simulink model "robot_kinematic_sim"  (R2020b)
settings: Solver=VariableStepAuto  StartTime=0.0  StopTime=10.0  RelTol=1e-3
model workspace (MAT data in the model file):
  dq0 = [0; 0; 0; 0; 0; 0]
  q0 = [0; 0; 0; 0; 0; 0]
  robotToTest : robotics.RigidBodyTree (value not decoded)
undefined symbols (not defined in the file): X, Y, Z
libraries: fl_lib x114, sm_lib x104, nesl_utility x59, fl_ps_legacy_lib x48, ee_lib x12, simulink_extras x10, simulink x6
(part 1 of 5)
settings Model: Creator=jeason  LastModifiedBy=Administrator
settings ExplorerConfigSet: InternalSimMechanicsExplorerSettings="<ExplorerConfiguration><canonicalview>default</canonicalview><AnimationSetting><BasePlaybackSpeed>1.0</BasePlaybackSpeed><PlaybackSpeedFactor>1.0</PlaybackSpeedFactor></AnimationSetting><TilePanel><TileInfo><Tiles Columns=\"1\" Count=\"1\" Rows=\"1\"><Column Weight=\"1\"/><Row Weight=\"1\"/><Tile Height=\"1\" Width=\"1\" X=\"0\" Y=\"0\"/></Tiles></TileInfo><SceneInfo><SceneViewer><BGColor><R>0.800000011920928955</R><G>0.800000011920928955</G><B>0.800000011920928955</B></BGColor><Camera><DoPerspective>off</DoPerspective><Position><X>0.99999999731974798</X><Y>-0.0550754879096279348</Y><Z>0.380291677713366028</Z></Position><Center><X>-2.68025202032617926e-09</X><Y>-0.0550754879096279348</Y><Z>0.380291677713366028</Z></Center><Up><X>-0</X><Y>0</Y><Z>1</Z></Up><ViewLimDist>1</ViewLimDist><YLim>0.484052245622873412</YLim><XLim>0.721172433512456745</XLim></Camera></SceneViewer></SceneInfo></TilePanel><MechEditorPanel><Cameras/><GfxPanelCameraMap/><VideoCreator><PlaybackSpeedRatio>1.0</PlaybackSpeedRatio><FrameRate>30</FrameRate><VideoFormat>Motion JPEG AVI</VideoFormat><FrameSize>auto</FrameSize></VideoCreator></MechEditorPanel></ExplorerConfiguration>"

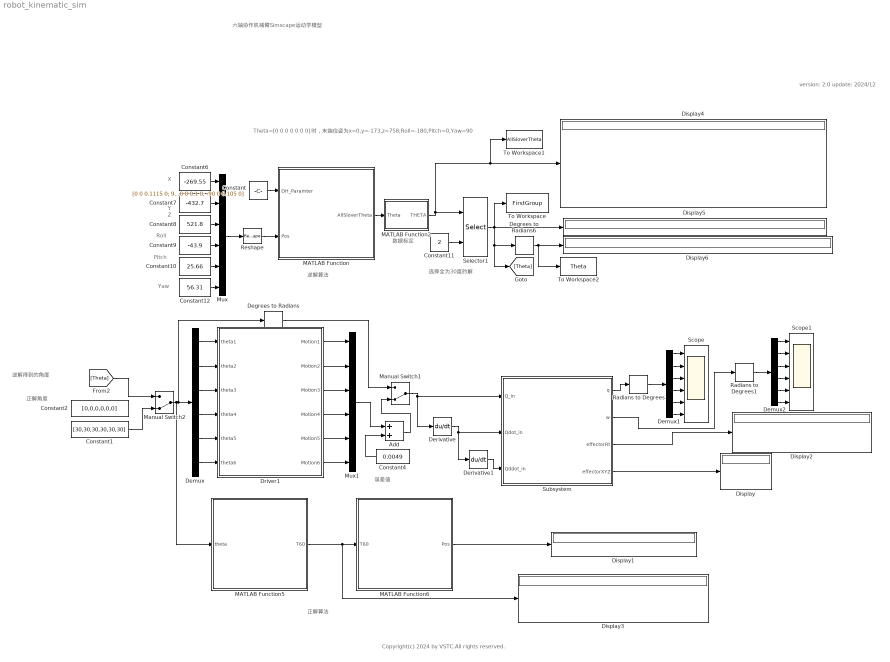
== system robot_kinematic_sim ==
  note: 六轴协作机械臂Simscape运动学模型
  note: Copyright(c) 2024 by VSTC.All rights reserved.
  note: Y
  note: Pitch
  note: X
  note: Theta=[0 0 0 0 0 0 0]时，末端位姿为x=0,y=-173,z=758;Roll=-180,Pitch=0,Yaw=90
  note: 数据标定
  note: Z
  note: 逆解算法
  note: Roll
  note: 选择全为30度的解
  note: Yaw
  note: 逆解得到的角度
  note: 正解角度
  note: 正解算法
  note: 误差值
  note: version: 2.0
      | update: 2024/12
  Add : Sum
  Constant : Constant  Value=[0 0 0.1115 0; 90 0 0 -90;0 -0.27 0 0; 0 -0.248 0.149 -90; 90 0 0.1 0; -90 0 0.105 0]
  Constant1 : Constant  Value=[30,30,30,30,30,30]
  Constant10 : Constant  Value=25.66
  Constant11 : Constant  Value=2
  Constant12 : Constant  Value=56.31
  Constant2 : Constant  Value=[0,0,0,0,0,0]
  Constant4 : Constant  Value=0.0049
  Constant6 : Constant  Value=-269.55
  Constant7 : Constant  Value=-432.7
  Constant8 : Constant  Value=521.8
  Constant9 : Constant  Value=-43.9
  Degrees to Radians : simulink_extras/Transformations/Degrees to Radians
  Degrees to Radians6 : simulink_extras/Transformations/Degrees to Radians
  Demux : Demux  Outputs=6
  Demux1 : Demux  Outputs=6
  Demux2 : Demux  Outputs=6
  Derivative : Derivative
  Derivative1 : Derivative
  Display{,1..6} (7 alike) : Display
  Driver1 : Subsystem  mask{angle1=0, angle2=0, angle3=0, angle4=0, angle5=0, angle6=0}
      mask prompts: angle1 "InitTheta1(deg)"; angle2 "InitTheta2(deg)"; angle3 "InitTheta3(deg)"; angle4 "InitTheta4(deg)"; angle5 "InitTheta5(deg)"; angle6 "InitTheta6(deg)"
  MATLAB Function : MATLAB Function  TreatAsAtomicUnit=on
  MATLAB Function2 : MATLAB Function  TreatAsAtomicUnit=on
  MATLAB Function5 : MATLAB Function  TreatAsAtomicUnit=on
  MATLAB Function6 : MATLAB Function  TreatAsAtomicUnit=on
  Manual Switch1 : ManualSwitch  CurrentSetting=0
  Manual Switch2 : ManualSwitch  CurrentSetting=0
  Mux : Mux  Inputs=6
  Mux1 : Mux  Inputs=6
  Radians to Degrees : simulink_extras/Transformations/Radians to Degrees
  Radians to Degrees1 : simulink_extras/Transformations/Radians to Degrees
  Reshape : Reshape  OutputDimensionality=Customize  OutputDimensions=[1,6]
  Scope : Scope  NumInputPorts=6
  Scope1 : Scope  NumInputPorts=6
  Selector1 : Selector  NumberOfDimensions=2  InputPortWidth=10  IndexOptions=Index vector (port),Starting index (dialog)  Indices=[1 3],1  OutputSizes=1,6
  Subsystem : Subsystem
  To Workspace : ToWorkspace  VariableName=FirstGroup  SampleTime=-1
      settings: MaxDataPoints=1
  To Workspace1 : ToWorkspace  VariableName=AllSloverTheta  SampleTime=-1
      settings: MaxDataPoints=1
  To Workspace2 : ToWorkspace  VariableName=Theta  SampleTime=-1
      settings: MaxDataPoints=1
  wires:
  Constant4/1 -> Add/2
  Degrees to Radians/1 -> Manual Switch1/1
  Driver1/6(Motion6) -> Mux1/6
  Manual Switch1/1 -> Derivative/1, Subsystem/1(Q_in)
  Demux1/6 -> Scope/6
  Driver1/5(Motion5) -> Mux1/5
  Degrees to Radians6/1 -> Display6/1, To Workspace2/1
  Demux/3 -> Driver1/3(theta3)
  Driver1/4(Motion4) -> Mux1/4
  Derivative1/1 -> Subsystem/3(Qddot_in)
  Constant12/1 -> Mux/6
  Demux/6 -> Driver1/6(theta6)
  Demux/4 -> Driver1/4(theta4)
  Driver1/3(Motion3) -> Mux1/3
  Driver1/2(Motion2) -> Mux1/2
  Constant/1 -> MATLAB Function/1(DH_Paramter)
  Demux1/1 -> Scope/1
  Driver1/1(Motion1) -> Mux1/1
  [Theta] -> Manual Switch2/1
  MATLAB Function6/1(Pos) -> Display1/1
  Demux/5 -> Driver1/5(theta5)
  Constant8/1 -> Mux/3
  Constant11/1 -> Selector1/2
  Demux/1 -> Driver1/1(theta1)
  MATLAB Function/1(AllSloverTheta) -> MATLAB Function2/1(Theta)
  Demux1/5 -> Scope/5
  Derivative/1 -> Derivative1/1, Subsystem/2(Qdot_in)
  Constant10/1 -> Mux/5
  Reshape/1 -> MATLAB Function/2(Pos)
  Demux1/3 -> Scope/3
  Constant7/1 -> Mux/2
  MATLAB Function2/1(THETA) -> Selector1/1, Display4/1, To Workspace1/1
  Constant6/1 -> Mux/1
  Constant9/1 -> Mux/4
  Selector1/1 -> Display5/1, Degrees to Radians6/1, [Theta], To Workspace/1
  Manual Switch2/1 -> MATLAB Function5/1(theta), Degrees to Radians/1, Demux/1
  Mux/1 -> Reshape/1
  Subsystem/4(effectorXYZ) -> Display/1
  Radians to Degrees/1 -> Demux1/1
  Demux1/4 -> Scope/4
  Subsystem/3(effectorRt) -> Display2/1
  Demux1/2 -> Scope/2
  Demux/2 -> Driver1/2(theta2)
  Demux2/2 -> Scope1/2
  Demux2/4 -> Scope1/4
  Radians to Degrees1/1 -> Demux2/1
  Demux2/3 -> Scope1/3
  Demux2/1 -> Scope1/1
  Subsystem/2(w) -> Radians to Degrees1/1
  Demux2/5 -> Scope1/5
  Demux2/6 -> Scope1/6
  Mux1/1 -> Add/1
  Add/1 -> Manual Switch1/2
  Subsystem/1(q) -> Radians to Degrees/1
  MATLAB Function5/1(T60) -> Display3/1, MATLAB Function6/1(T60)
  Constant1/1 -> Manual Switch2/2

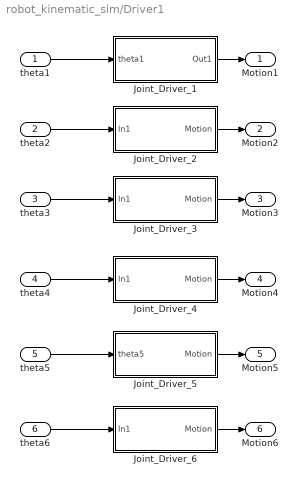
== system robot_kinematic_sim/Driver1 ==
  theta1 : Inport
  theta2 : Inport  Port=2
  theta3 : Inport  Port=3
  theta4 : Inport  Port=4
  theta5 : Inport  Port=5
  theta6 : Inport  Port=6
  Joint_Driver_1 : Subsystem
  Joint_Driver_2 : Subsystem
  Joint_Driver_3 : Subsystem
  Joint_Driver_4 : Subsystem
  Joint_Driver_5 : Subsystem
  Joint_Driver_6 : Subsystem
  Motion1 : Outport
  Motion2 : Outport  Port=2
  Motion3 : Outport  Port=3
  Motion4 : Outport  Port=4
  Motion5 : Outport  Port=5
  Motion6 : Outport  Port=6
  wires:
  theta2/1 -> Joint_Driver_2/1(In1)
  theta3/1 -> Joint_Driver_3/1(In1)
  theta4/1 -> Joint_Driver_4/1(In1)
  theta6/1 -> Joint_Driver_6/1(In1)
  theta1/1 -> Joint_Driver_1/1(theta1)
  Joint_Driver_1/1(Out1) -> Motion1/1
  Joint_Driver_2/1(Motion) -> Motion2/1
  Joint_Driver_3/1(Motion) -> Motion3/1
  Joint_Driver_4/1(Motion) -> Motion4/1
  Joint_Driver_5/1(Motion) -> Motion5/1
  Joint_Driver_6/1(Motion) -> Motion6/1
  theta5/1 -> Joint_Driver_5/1(theta5)

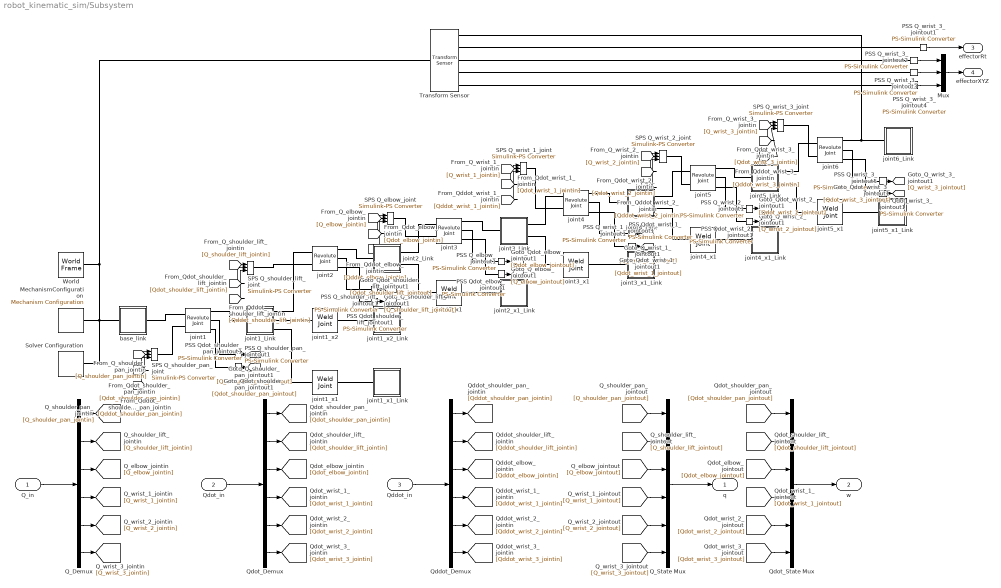
== system robot_kinematic_sim/Subsystem ==
  Q_in : Inport
  Qdot_in : Inport  Port=2
  Qddot_in : Inport  Port=3
  MechanismConfiguration : sm_lib/Utilities/Mechanism Configuration  UniformGravity=Constant  GravityVector=[0 0 -9.80665]  GravityVectorUnits=m/s^2  GravityUnits=m/s^2  LinearizationDelta=0.001  JointModeTransitionNonlinearIterations=2
  Mux : Mux  Inputs=3
  PSS Q_elbow_jointout1 : nesl_utility/PS-Simulink Converter  PhysicalDomain=network_engine_domain  SubClassName=ps_output  LeftPortType=input  RightPortType=output  PseudoPeriodic=off  Frequencies=[]  Unit=inherit  AffineConversion=off
  PSS Q_shoulder_lift_jointout1 : nesl_utility/PS-Simulink Converter  PhysicalDomain=network_engine_domain  SubClassName=ps_output  LeftPortType=input  RightPortType=output  PseudoPeriodic=off  Frequencies=[]  Unit=inherit  AffineConversion=off
  PSS Q_shoulder_pan_jointout1 : nesl_utility/PS-Simulink Converter  PhysicalDomain=network_engine_domain  SubClassName=ps_output  LeftPortType=input  RightPortType=output  PseudoPeriodic=off  Frequencies=[]  Unit=inherit  AffineConversion=off
  PSS Q_wrist_1_jointout1 : nesl_utility/PS-Simulink Converter  PhysicalDomain=network_engine_domain  SubClassName=ps_output  LeftPortType=input  RightPortType=output  PseudoPeriodic=off  Frequencies=[]  Unit=inherit  AffineConversion=off
  PSS Q_wrist_2_jointout1 : nesl_utility/PS-Simulink Converter  PhysicalDomain=network_engine_domain  SubClassName=ps_output  LeftPortType=input  RightPortType=output  PseudoPeriodic=off  Frequencies=[]  Unit=inherit  AffineConversion=off
  PSS Q_wrist_3_jointout{1..5} (5 alike) : nesl_utility/PS-Simulink Converter  PhysicalDomain=network_engine_domain  SubClassName=ps_output  LeftPortType=input  RightPortType=output  PseudoPeriodic=off  Frequencies=[]  Unit=inherit  AffineConversion=off
  PSS Qdot_elbow_jointout1 : nesl_utility/PS-Simulink Converter  PhysicalDomain=network_engine_domain  SubClassName=ps_output  LeftPortType=input  RightPortType=output  PseudoPeriodic=off  Frequencies=[]  Unit=inherit  AffineConversion=off
  PSS Qdot_shoulder_lift_jointout1 : nesl_utility/PS-Simulink Converter  PhysicalDomain=network_engine_domain  SubClassName=ps_output  LeftPortType=input  RightPortType=output  PseudoPeriodic=off  Frequencies=[]  Unit=inherit  AffineConversion=off
  PSS Qdot_shoulder_pan_jointout1 : nesl_utility/PS-Simulink Converter  PhysicalDomain=network_engine_domain  SubClassName=ps_output  LeftPortType=input  RightPortType=output  PseudoPeriodic=off  Frequencies=[]  Unit=inherit  AffineConversion=off
  PSS Qdot_wrist_1_jointout1 : nesl_utility/PS-Simulink Converter  PhysicalDomain=network_engine_domain  SubClassName=ps_output  LeftPortType=input  RightPortType=output  PseudoPeriodic=off  Frequencies=[]  Unit=inherit  AffineConversion=off
  PSS Qdot_wrist_2_jointout1 : nesl_utility/PS-Simulink Converter  PhysicalDomain=network_engine_domain  SubClassName=ps_output  LeftPortType=input  RightPortType=output  PseudoPeriodic=off  Frequencies=[]  Unit=inherit  AffineConversion=off
  PSS Qdot_wrist_3_jointout1 : nesl_utility/PS-Simulink Converter  PhysicalDomain=network_engine_domain  SubClassName=ps_output  LeftPortType=input  RightPortType=output  PseudoPeriodic=off  Frequencies=[]  Unit=inherit  AffineConversion=off
  Q_Demux : Demux  Outputs=6
  Q_State Mux : Mux  Inputs=6
  Qddot_Demux : Demux  Outputs=6
  Qdot_Demux : Demux  Outputs=6
  Qdot_State Mux : Mux  Inputs=6
  SPS Q_elbow_joint : nesl_utility/Simulink-PS Converter  PhysicalDomain=network_engine_domain  SubClassName=ps_input  LeftPortType=input  RightPortType=output  PseudoPeriodic=off  Frequencies=[]  NoiseDistribution=none  NoiseParameters=[]  Unit=1  AffineConversion=off  FilteringAndDerivatives=provide  SimscapeFilterOrder=1  InputFilterTimeConstant=0.001  UdotUserProvided=2
  SPS Q_shoulder_lift_joint : nesl_utility/Simulink-PS Converter  PhysicalDomain=network_engine_domain  SubClassName=ps_input  LeftPortType=input  RightPortType=output  PseudoPeriodic=off  Frequencies=[]  NoiseDistribution=none  NoiseParameters=[]  Unit=1  AffineConversion=off  FilteringAndDerivatives=provide  SimscapeFilterOrder=1  InputFilterTimeConstant=0.001  UdotUserProvided=2
  SPS Q_shoulder_pan_joint : nesl_utility/Simulink-PS Converter  PhysicalDomain=network_engine_domain  SubClassName=ps_input  LeftPortType=input  RightPortType=output  PseudoPeriodic=off  Frequencies=[]  NoiseDistribution=none  NoiseParameters=[]  Unit=1  AffineConversion=off  FilteringAndDerivatives=provide  SimscapeFilterOrder=1  InputFilterTimeConstant=0.001  UdotUserProvided=2
  SPS Q_wrist_1_joint : nesl_utility/Simulink-PS Converter  PhysicalDomain=network_engine_domain  SubClassName=ps_input  LeftPortType=input  RightPortType=output  PseudoPeriodic=off  Frequencies=[]  NoiseDistribution=none  NoiseParameters=[]  Unit=1  AffineConversion=off  FilteringAndDerivatives=provide  SimscapeFilterOrder=1  InputFilterTimeConstant=0.001  UdotUserProvided=2
  SPS Q_wrist_2_joint : nesl_utility/Simulink-PS Converter  PhysicalDomain=network_engine_domain  SubClassName=ps_input  LeftPortType=input  RightPortType=output  PseudoPeriodic=off  Frequencies=[]  NoiseDistribution=none  NoiseParameters=[]  Unit=1  AffineConversion=off  FilteringAndDerivatives=provide  SimscapeFilterOrder=1  InputFilterTimeConstant=0.001  UdotUserProvided=2
  SPS Q_wrist_3_joint : nesl_utility/Simulink-PS Converter  PhysicalDomain=network_engine_domain  SubClassName=ps_input  LeftPortType=input  RightPortType=output  PseudoPeriodic=off  Frequencies=[]  NoiseDistribution=none  NoiseParameters=[]  Unit=1  AffineConversion=off  FilteringAndDerivatives=provide  SimscapeFilterOrder=1  InputFilterTimeConstant=0.001  UdotUserProvided=2
  Solver Configuration : nesl_utility/Solver Configuration  PhysicalDomain=network_engine_domain  SubClassName=solver  LeftPortType=input  RightPortType=generic  Tones=[]  Harmonics=[]  SimulateNoise=off  Profile=off  UseLocalSampling=off  EnableSwitchedLinearOptims=on  FrequencyDomain=off  UseCCode=off  RelTol=0.001  AbsTol=1e-06  MinStep=1e-09  ToWorkspace=off  RevertToSquareIcSolve=off  NumHomotopyIterations=100  PhasorMode=off  PhasorModeNumHarmonics=1  DoDC=off  ResidualTolerance=1e-09  UseLocalSolver=off  LocalSolverChoice=NE_BACKWARD_EULER_ADVANCER  LocalSolverSampleTime=0.001  DoFixedCost=off  MaxNonlinIter=3  MaxModeIter=2  ComputeImpulses=off  ImpulseIterations=2  LinearAlgebra=auto  EquationFormulation=NE_TIME_EF  DelaysMemoryBudget=1024  AutomaticFiltering=on  FilteringTimeConstant=0.001  PartitionStorageMethod=AS_NEEDED  PartitionMemoryBudget=1024  PartitionMethod=ROBUST
  Transform Sensor : sm_lib/Frames and Transforms/Transform Sensor  MeasurementFrame=Base  SenseAngle=off  SenseAxis=off  SenseAngle1=off  SenseAngle2=off  SenseAngle3=off  SenseQ=off  SenseR=on  SenseOmegaX=off  SenseOmegaY=off  SenseOmegaZ=off  SenseOmega1=off  SenseOmega2=off  SenseOmega3=off  SenseQDot=off  SenseRDot=off  SenseAlphaX=off  SenseAlphaY=off  SenseAlphaZ=off  SenseAlpha1=off  SenseAlpha2=off  SenseAlpha3=off  SenseQDDot=off  SenseRDDot=off  SenseX=on  SenseY=on  SenseZ=on  SenseRad=off  SenseAzim=off  SenseDist=off  SenseInc=off  SenseXDot=off  SenseYDot=off  SenseZDot=off  SenseRadDot=off  SenseAzimDot=off  SenseDistDot=off  SenseIncDot=off  SenseXDDot=off  SenseYDDot=off  SenseZDDot=off  SenseRadDDot=off  SenseAzimDDot=off  SenseDistDDot=off  SenseIncDDot=off
  World : sm_lib/Frames and Transforms/World Frame
  base_link : Subsystem
  joint{1..6} (6 alike) : sm_lib/Joints/Revolute Joint  JointMode=Normal  SenseConstraintForce=off  SenseConstraintForceX=off  SenseConstraintForceY=off  SenseConstraintForceZ=off  SenseConstraintTorque=off  SenseConstraintTorqueX=off  SenseConstraintTorqueY=off  SenseConstraintTorqueZ=off  SenseTotalForce=off  SenseTotalForceX=off  SenseTotalForceY=off  SenseTotalForceZ=off  SenseTotalTorque=off  SenseTotalTorqueX=off  SenseTotalTorqueY=off  SenseTotalTorqueZ=off  CompositeWrenchDir=FollowerOnBase  CompositeWrenchFrame=BaseFrame  EquilibriumPosition=0  EquilibriumPositionUnits=deg  SpringStiffness=0  SpringStiffnessUnits=N*m/deg  DampingCoefficient=0  DampingCoefficientUnits=m^2*kg/s/rad  TorqueActuationMode=ComputedTorque  MotionActuationMode=InputMotion  SensePosition=on  SenseVelocity=on  SenseAcceleration=off  SenseTorqueForce=off  SenseLowerLimitTorqueForce=off  SenseUpperLimitTorqueForce=off  LowerLimitSpecify=on  LowerLimitBound=-3.1415  LowerLimitBoundUnits=rad  LowerLimitStiffness=1e4  LowerLimitStiffnessUnits=N*m/deg  LowerLimitDamping=10  LowerLimitDampingUnits=N*m/(deg/s)  LowerLimitTransitionRegionWidth=0.1  LowerLimitTransitionRegionWidthUnits=deg  UpperLimitSpecify=on  UpperLimitBound=3.1415  UpperLimitBoundUnits=rad  UpperLimitStiffness=1e4  UpperLimitStiffnessUnits=N*m/deg  UpperLimitDamping=10  UpperLimitDampingUnits=N*m/(deg/s)  UpperLimitTransitionRegionWidth=0.1  UpperLimitTransitionRegionWidthUnits=deg  PositionTargetSpecify=off  PositionTargetPriority=High  PositionTargetValue=0  PositionTargetValueUnits=deg  VelocityTargetSpecify=off  VelocityTargetPriority=High  VelocityTargetValue=0  VelocityTargetValueUnits=deg/s
  joint1_Link : Subsystem
  joint1_x1 : sm_lib/Joints/Weld Joint  JointMode=Normal  SenseConstraintForce=off  SenseConstraintForceX=off  SenseConstraintForceY=off  SenseConstraintForceZ=off  SenseConstraintTorque=off  SenseConstraintTorqueX=off  SenseConstraintTorqueY=off  SenseConstraintTorqueZ=off  SenseTotalForce=off  SenseTotalForceX=off  SenseTotalForceY=off  SenseTotalForceZ=off  SenseTotalTorque=off  SenseTotalTorqueX=off  SenseTotalTorqueY=off  SenseTotalTorqueZ=off  CompositeWrenchDir=FollowerOnBase  CompositeWrenchFrame=BaseFrame
  joint1_x1_Link : Subsystem
  joint1_x2 : sm_lib/Joints/Weld Joint  JointMode=Normal  SenseConstraintForce=off  SenseConstraintForceX=off  SenseConstraintForceY=off  SenseConstraintForceZ=off  SenseConstraintTorque=off  SenseConstraintTorqueX=off  SenseConstraintTorqueY=off  SenseConstraintTorqueZ=off  SenseTotalForce=off  SenseTotalForceX=off  SenseTotalForceY=off  SenseTotalForceZ=off  SenseTotalTorque=off  SenseTotalTorqueX=off  SenseTotalTorqueY=off  SenseTotalTorqueZ=off  CompositeWrenchDir=FollowerOnBase  CompositeWrenchFrame=BaseFrame
  joint1_x2_Link : Subsystem
  joint2_Link : Subsystem
  joint2_x1 : sm_lib/Joints/Weld Joint  JointMode=Normal  SenseConstraintForce=off  SenseConstraintForceX=off  SenseConstraintForceY=off  SenseConstraintForceZ=off  SenseConstraintTorque=off  SenseConstraintTorqueX=off  SenseConstraintTorqueY=off  SenseConstraintTorqueZ=off  SenseTotalForce=off  SenseTotalForceX=off  SenseTotalForceY=off  SenseTotalForceZ=off  SenseTotalTorque=off  SenseTotalTorqueX=off  SenseTotalTorqueY=off  SenseTotalTorqueZ=off  CompositeWrenchDir=FollowerOnBase  CompositeWrenchFrame=BaseFrame
  joint2_x1_Link : Subsystem
  joint3_Link : Subsystem
  joint3_x1 : sm_lib/Joints/Weld Joint  JointMode=Normal  SenseConstraintForce=off  SenseConstraintForceX=off  SenseConstraintForceY=off  SenseConstraintForceZ=off  SenseConstraintTorque=off  SenseConstraintTorqueX=off  SenseConstraintTorqueY=off  SenseConstraintTorqueZ=off  SenseTotalForce=off  SenseTotalForceX=off  SenseTotalForceY=off  SenseTotalForceZ=off  SenseTotalTorque=off  SenseTotalTorqueX=off  SenseTotalTorqueY=off  SenseTotalTorqueZ=off  CompositeWrenchDir=FollowerOnBase  CompositeWrenchFrame=BaseFrame
  joint3_x1_Link : Subsystem
  joint4_Link : Subsystem
  joint4_x1 : sm_lib/Joints/Weld Joint  JointMode=Normal  SenseConstraintForce=off  SenseConstraintForceX=off  SenseConstraintForceY=off  SenseConstraintForceZ=off  SenseConstraintTorque=off  SenseConstraintTorqueX=off  SenseConstraintTorqueY=off  SenseConstraintTorqueZ=off  SenseTotalForce=off  SenseTotalForceX=off  SenseTotalForceY=off  SenseTotalForceZ=off  SenseTotalTorque=off  SenseTotalTorqueX=off  SenseTotalTorqueY=off  SenseTotalTorqueZ=off  CompositeWrenchDir=FollowerOnBase  CompositeWrenchFrame=BaseFrame
  joint4_x1_Link : Subsystem
  joint5_Link : Subsystem
  joint5_x1 : sm_lib/Joints/Weld Joint  JointMode=Normal  SenseConstraintForce=off  SenseConstraintForceX=off  SenseConstraintForceY=off  SenseConstraintForceZ=off  SenseConstraintTorque=off  SenseConstraintTorqueX=off  SenseConstraintTorqueY=off  SenseConstraintTorqueZ=off  SenseTotalForce=off  SenseTotalForceX=off  SenseTotalForceY=off  SenseTotalForceZ=off  SenseTotalTorque=off  SenseTotalTorqueX=off  SenseTotalTorqueY=off  SenseTotalTorqueZ=off  CompositeWrenchDir=FollowerOnBase  CompositeWrenchFrame=BaseFrame
  joint5_x1_Link : Subsystem
  joint6_Link : Subsystem
  q : Outport
  w : Outport  Port=2
  effectorRt : Outport  Port=3
  effectorXYZ : Outport  Port=4
  wires:
  [Q_elbow_jointin] -> SPS Q_elbow_joint/1
  Q_Demux/4 -> [Q_wrist_1_jointin]
  [Q_wrist_3_jointout] -> Q_State Mux/6
  [Qdot_elbow_jointin] -> SPS Q_elbow_joint/2
  [Qdot_wrist_2_jointout] -> Qdot_State Mux/5
  [Qdot_shoulder_lift_jointout] -> Qdot_State Mux/2
  Q_in/1 -> Q_Demux/1
  [Qdot_shoulder_pan_jointin] -> SPS Q_shoulder_pan_joint/2
  [Qdot_wrist_1_jointout] -> Qdot_State Mux/4
  Q_Demux/6 -> [Q_wrist_3_jointin]
  Qdot_Demux/2 -> [Qdot_shoulder_lift_jointin]
  [Q_shoulder_pan_jointout] -> Q_State Mux/1
  [Qddot_elbow_jointin] -> SPS Q_elbow_joint/3
  [Q_shoulder_pan_jointin] -> SPS Q_shoulder_pan_joint/1
  [Q_wrist_2_jointin] -> SPS Q_wrist_2_joint/1
  Q_Demux/2 -> [Q_shoulder_lift_jointin]
  Qdot_Demux/4 -> [Qdot_wrist_1_jointin]
  Qddot_in/1 -> Qddot_Demux/1
  [Qddot_shoulder_pan_jointin] -> SPS Q_shoulder_pan_joint/3
  [Qddot_shoulder_lift_jointin] -> SPS Q_shoulder_lift_joint/3
  [Qddot_wrist_2_jointin] -> SPS Q_wrist_2_joint/3
  Qddot_Demux/3 -> [Qddot_elbow_jointin]
  [Qddot_wrist_1_jointin] -> SPS Q_wrist_1_joint/3
  [Q_shoulder_lift_jointout] -> Q_State Mux/2
  [Q_wrist_1_jointout] -> Q_State Mux/4
  [Qdot_shoulder_lift_jointin] -> SPS Q_shoulder_lift_joint/2
  Qdot_Demux/3 -> [Qdot_elbow_jointin]
  Qdot_in/1 -> Qdot_Demux/1
  Qddot_Demux/1 -> [Qddot_shoulder_pan_jointin]
  Qdot_Demux/6 -> [Qdot_wrist_3_jointin]
  Qdot_Demux/1 -> [Qdot_shoulder_pan_jointin]
  Qddot_Demux/6 -> [Qddot_wrist_3_jointin]
  [Q_elbow_jointout] -> Q_State Mux/3
  Qddot_Demux/2 -> [Qddot_shoulder_lift_jointin]
  Qdot_Demux/5 -> [Qdot_wrist_2_jointin]
  [Qdot_wrist_2_jointin] -> SPS Q_wrist_2_joint/2
  [Q_wrist_1_jointin] -> SPS Q_wrist_1_joint/1
  [Qdot_wrist_1_jointin] -> SPS Q_wrist_1_joint/2
  Qddot_Demux/4 -> [Qddot_wrist_1_jointin]
  [Qdot_wrist_3_jointout] -> Qdot_State Mux/6
  Qddot_Demux/5 -> [Qddot_wrist_2_jointin]
  Q_Demux/5 -> [Q_wrist_2_jointin]
  [Qdot_elbow_jointout] -> Qdot_State Mux/3
  Qdot_State Mux/1 -> w/1
  [Q_wrist_2_jointout] -> Q_State Mux/5
  Q_State Mux/1 -> q/1
  [Qdot_shoulder_pan_jointout] -> Qdot_State Mux/1
  [Q_wrist_3_jointin] -> SPS Q_wrist_3_joint/1
  [Qdot_wrist_3_jointin] -> SPS Q_wrist_3_joint/2
  [Qddot_wrist_3_jointin] -> SPS Q_wrist_3_joint/3
  Q_Demux/3 -> [Q_elbow_jointin]
  Mux/1 -> effectorXYZ/1
  PSS Q_wrist_3_jointout3/1 -> Mux/2
  PSS Q_wrist_3_jointout2/1 -> Mux/1
  PSS Q_wrist_3_jointout5/1 -> [Q_wrist_3_jointout]
  PSS Q_wrist_1_jointout1/1 -> [Q_wrist_1_jointout]
  PSS Q_shoulder_lift_jointout1/1 -> [Q_shoulder_lift_jointout]
  PSS Q_wrist_3_jointout1/1 -> effectorRt/1
  PSS Qdot_shoulder_lift_jointout1/1 -> [Qdot_shoulder_lift_jointout]
  PSS Qdot_wrist_3_jointout1/1 -> [Qdot_wrist_3_jointout]
  PSS Q_shoulder_pan_jointout1/1 -> [Q_shoulder_pan_jointout]
  PSS Qdot_shoulder_pan_jointout1/1 -> [Qdot_shoulder_pan_jointout]
  PSS Q_elbow_jointout1/1 -> [Q_elbow_jointout]
  PSS Q_wrist_2_jointout1/1 -> [Q_wrist_2_jointout]
  PSS Qdot_wrist_2_jointout1/1 -> [Qdot_wrist_2_jointout]
  PSS Q_wrist_3_jointout4/1 -> Mux/3
  PSS Qdot_wrist_1_jointout1/1 -> [Qdot_wrist_1_jointout]
  PSS Qdot_elbow_jointout1/1 -> [Qdot_elbow_jointout]
  [Q_shoulder_lift_jointin] -> SPS Q_shoulder_lift_joint/1
  Q_Demux/1 -> [Q_shoulder_pan_jointin]
  connections:
  Transform Sensor/RConn2 -- PSS Q_wrist_3_jointout1/LConn1
  PSS Q_wrist_3_jointout2/LConn1 -- Transform Sensor/RConn3
  Transform Sensor/RConn5 -- PSS Q_wrist_3_jointout4/LConn1
  Transform Sensor/RConn4 -- PSS Q_wrist_3_jointout3/LConn1
  base_link/LConn1 -- MechanismConfiguration/RConn1 -- Solver Configuration/RConn1 -- Transform Sensor/LConn1 -- World/RConn1
  joint4_Link/RConn2 -- joint4_x1/LConn1
  joint1/RConn1 -- joint1_Link/LConn1
  joint2_Link/RConn2 -- joint2_x1/LConn1
  joint3_x1/RConn1 -- joint3_x1_Link/LConn1
  joint2/RConn1 -- joint2_Link/LConn1
  joint5_x1/RConn1 -- joint5_x1_Link/LConn1
  joint1_x1/RConn1 -- joint1_x1_Link/LConn1
  joint1_Link/RConn1 -- joint2/LConn1
  joint1_x2/RConn1 -- joint1_x2_Link/LConn1
  joint5_Link/RConn1 -- joint6/LConn1
  joint6/RConn1 -- Transform Sensor/RConn1 -- joint6_Link/LConn1
  joint5_Link/RConn2 -- joint5_x1/LConn1
  joint5/RConn1 -- joint5_Link/LConn1
  joint4_Link/RConn1 -- joint5/LConn1
  base_link/RConn1 -- joint1/LConn1
  joint3_Link/RConn2 -- joint3_x1/LConn1
  joint1_Link/RConn2 -- joint1_x2/LConn1
  joint4_x1/RConn1 -- joint4_x1_Link/LConn1
  joint1_Link/RConn3 -- joint1_x1/LConn1
  joint4/RConn1 -- joint4_Link/LConn1
  joint2_Link/RConn1 -- joint3/LConn1
  joint2_x1/RConn1 -- joint2_x1_Link/LConn1
  joint3/RConn1 -- joint3_Link/LConn1
  joint3_Link/RConn1 -- joint4/LConn1
  SPS Q_shoulder_pan_joint/RConn1 -- joint1/LConn2
  PSS Q_shoulder_pan_jointout1/LConn1 -- joint1/RConn2
  PSS Qdot_shoulder_pan_jointout1/LConn1 -- joint1/RConn3
  SPS Q_shoulder_lift_joint/RConn1 -- joint2/LConn2
  PSS Q_shoulder_lift_jointout1/LConn1 -- joint2/RConn2
  joint2/RConn3 -- PSS Qdot_shoulder_lift_jointout1/LConn1
  joint3/LConn2 -- SPS Q_elbow_joint/RConn1
  PSS Q_elbow_jointout1/LConn1 -- joint3/RConn2
  PSS Qdot_elbow_jointout1/LConn1 -- joint3/RConn3
  SPS Q_wrist_1_joint/RConn1 -- joint4/LConn2
  PSS Q_wrist_1_jointout1/LConn1 -- joint4/RConn2
  PSS Qdot_wrist_1_jointout1/LConn1 -- joint4/RConn3
  SPS Q_wrist_2_joint/RConn1 -- joint5/LConn2
  PSS Q_wrist_2_jointout1/LConn1 -- joint5/RConn2
  PSS Qdot_wrist_2_jointout1/LConn1 -- joint5/RConn3
  SPS Q_wrist_3_joint/RConn1 -- joint6/LConn2
  PSS Q_wrist_3_jointout5/LConn1 -- joint6/RConn2
  joint6/RConn3 -- PSS Qdot_wrist_3_jointout1/LConn1

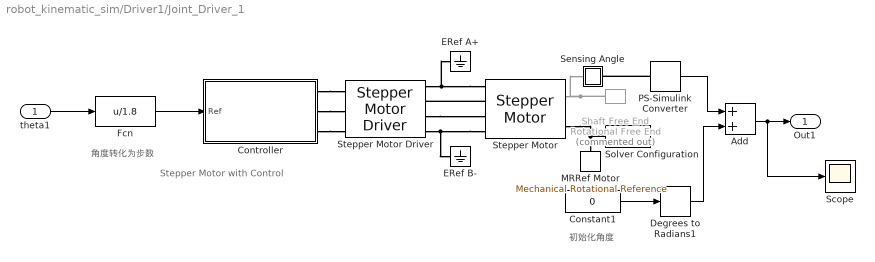
== system robot_kinematic_sim/Driver1/Joint_Driver_1 ==
  note: 初始化角度
  note: Stepper Motor with Control
  note: 角度转化为步数
  theta1 : Inport
  Add : Sum
  Constant1 : Constant  Value=0
  Controller : Subsystem  Variant=on  VariantControlMode=label  LabelModeActiveChoice=Position
      settings: AttributesFormatString=%<BlockChoice>
  Degrees to Radians1 : simulink_extras/Transformations/Degrees to Radians
  ERef A+ : fl_lib/Electrical/Electrical Elements/Electrical Reference
      settings: SourceProductName=Foundation Library
  ERef B- : fl_lib/Electrical/Electrical Elements/Electrical Reference
      settings: SourceProductName=Foundation Library
  Fcn : Fcn  Expr=u/1.8
  MRRef Motor : fl_lib/Mechanical/Rotational Elements/Mechanical Rotational Reference
      settings: SourceProductName=Foundation Library
  PS-Simulink Converter : nesl_utility/PS-Simulink Converter  PhysicalDomain=network_engine_domain  SubClassName=ps_output  LeftPortType=input  RightPortType=output  PseudoPeriodic=off  Frequencies=[]  Unit=1  AffineConversion=off
  Scope : Scope
  Sensing Angle : Subsystem
      mask Display: disp('a')
  Shaft Free End : fl_lib/Mechanical/Rotational Elements/Rotational Free End  Commented=on  w_specify=off  w_priority=None  w=0 rad/s  w_nominal_specify=off  w_nominal_unit=rad/s  w_nominal_value=1
      settings: SourceProductName=Foundation Library
  Solver Configuration : nesl_utility/Solver Configuration  PhysicalDomain=network_engine_domain  SubClassName=solver  LeftPortType=input  RightPortType=generic  Tones=[]  Harmonics=[]  SimulateNoise=off  Profile=off  UseLocalSampling=off  EnableSwitchedLinearOptims=on  FrequencyDomain=off  UseCCode=off  RelTol=0.001  AbsTol=1e-06  MinStep=1e-09  ToWorkspace=off  RevertToSquareIcSolve=off  NumHomotopyIterations=100  PhasorMode=off  PhasorModeNumHarmonics=1  DoDC=off  ResidualTolerance=1e-9  UseLocalSolver=off  LocalSolverChoice=NE_BACKWARD_EULER_ADVANCER  LocalSolverSampleTime=.001  DoFixedCost=off  MaxNonlinIter=3  MaxModeIter=2  ComputeImpulses=off  ImpulseIterations=2  LinearAlgebra=Sparse  EquationFormulation=NE_TIME_EF  DelaysMemoryBudget=1024  AutomaticFiltering=on  FilteringTimeConstant=0.001  PartitionStorageMethod=AS_NEEDED  PartitionMemoryBudget=1024  PartitionMethod=FAST
  Stepper Motor : ee_lib/Electromechanical/Reluctance & Stepper/Stepper Motor  step_or_av=1  w_vec=[ 0 1000 3e+3 ] rpm  T_vec=[ 2 2 1 ] N*m  assert_if_slipping=0  J_total=1e-4 kg*m^2  max_step_rate=10 Hz  Ra=0.55 Ohm  La=0.0015 H  Km=0.19 N*m/A  Td=0 N*m  Rm=Inf Ohm  Step=1.8 deg  J=4.5e-05 kg*m^2  B=1e-02 N*m/(rad/s)  speed0=0 rpm  theta0=0 deg  alpha=[.00393, .00393] 1/K  Tmeas=25 degC  T_vec2=[1.7, 1.7, .85] N*m  Tmeas2=125 degC  half_stepping=0  thermal_masses=[100, 100] J/K  initial_temperatures=[25, 25] degC  rotor_thermal_mass=50 J/K  rotor_initial_temperature=25 degC  Rm_percent_rotor=90
      settings: SourceProductBaseCode=PS  InternalSimscapePortConfiguration="{\"Bottom\":[],\"Left\":[{\"id\":\"pA\",\"label\":\"A+\",\"type\":\"foundation.electrical.electrical\"},{\"id\":\"nA\",\"label\":\"A-\",\"type\":\"foundation.electrical.electrical\"},{\"id\":\"pB\",\"label\":\"B+\",\"type\":\"foundation.electrical.electrical\"},{\"id\":\"nB\",\"label\":\"B-\",\"type\":\"foundation.electrical.electrical\"}],\"Right\":[{\"id\":\"R\",\"label\":\"R\",\"type\":\"foundation.mechanical.rotational.rotational\"},{\"id\":\"C\",\"label\":\"C\",\"type\":\"foundation.mechanical.rotational.rotational\"}],\"Top\":[]}"
  Stepper Motor Driver : ee_lib/Electromechanical/Reluctance & Stepper/Stepper Motor Driver  step_or_av=1  step_rate_per_volt=10 Hz/V  Venable=2.5 V  Vreverse=2.5 V  Vm=5 V  half_stepping=0
      settings: SourceProductBaseCode=PS  InternalSimscapePortConfiguration="{\"Bottom\":[],\"Left\":[{\"id\":\"ENA\",\"label\":\"ENA\",\"type\":\"foundation.electrical.electrical\"},{\"id\":\"REF\",\"label\":\"REF\",\"type\":\"foundation.electrical.electrical\"},{\"id\":\"REV\",\"label\":\"REV\",\"type\":\"foundation.electrical.electrical\"}],\"Right\":[{\"id\":\"pA\",\"label\":\"A+\",\"type\":\"foundation.electrical.electrical\"},{\"id\":\"nA\",\"label\":\"A-\",\"type\":\"foundation.electrical.electrical\"},{\"id\":\"pB\",\"label\":\"B+\",\"type\":\"foundation.electrical.electrical\"},{\"id\":\"nB\",\"label\":\"B-\",\"type\":\"foundation.electrical.electrical\"}],\"Top\":[]}"
  Out1 : Outport
  wires:
  Fcn/1 -> Controller/1(Ref)
  theta1/1 -> Fcn/1
  PS-Simulink Converter/1 -> Add/1
  Add/1 -> Scope/1, Out1/1
  Constant1/1 -> Degrees to Radians1/1
  Degrees to Radians1/1 -> Add/2
  connections:
  PS-Simulink Converter/LConn1 -- Sensing Angle/RConn1
  Controller/RConn3 -- Stepper Motor Driver/LConn3
  Controller/RConn2 -- Stepper Motor Driver/LConn2
  Controller/RConn1 -- Stepper Motor Driver/LConn1
  Stepper Motor/LConn2 -- Stepper Motor Driver/RConn2
  Stepper Motor Driver/RConn3 -- Stepper Motor/LConn3
  Stepper Motor/LConn1 -- Stepper Motor Driver/RConn1 -- ERef A+/LConn1
  Solver Configuration/RConn1 -- Stepper Motor/RConn2 -- MRRef Motor/LConn1
  Shaft Free End/LConn1 -- Sensing Angle/LConn1 -- Stepper Motor/RConn1
  Stepper Motor/LConn4 -- Stepper Motor Driver/RConn4 -- ERef B-/LConn1

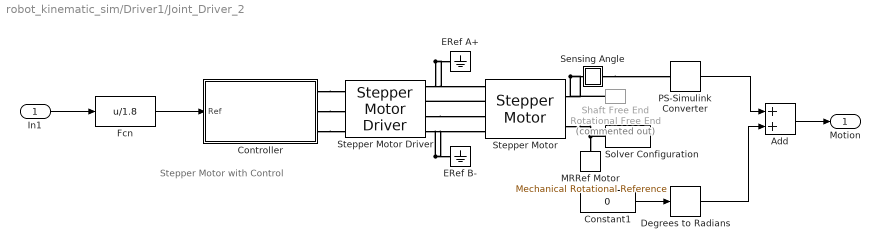
== system robot_kinematic_sim/Driver1/Joint_Driver_2 ==
  note: Stepper Motor with Control
  In1 : Inport
  Add : Sum
  Constant1 : Constant  Value=0
  Controller : Subsystem  Variant=on  VariantControlMode=label  LabelModeActiveChoice=Position
      settings: AttributesFormatString=%<BlockChoice>
  Degrees to Radians : simulink_extras/Transformations/Degrees to Radians
  ERef A+ : fl_lib/Electrical/Electrical Elements/Electrical Reference
      settings: SourceProductName=Foundation Library
  ERef B- : fl_lib/Electrical/Electrical Elements/Electrical Reference
      settings: SourceProductName=Foundation Library
  Fcn : Fcn  Expr=u/1.8
  MRRef Motor : fl_lib/Mechanical/Rotational Elements/Mechanical Rotational Reference
      settings: SourceProductName=Foundation Library
  PS-Simulink Converter : nesl_utility/PS-Simulink Converter  PhysicalDomain=network_engine_domain  SubClassName=ps_output  LeftPortType=input  RightPortType=output  PseudoPeriodic=off  Frequencies=[]  Unit=1  AffineConversion=off
  Sensing Angle : Subsystem
      mask Display: disp('a')
  Shaft Free End : fl_lib/Mechanical/Rotational Elements/Rotational Free End  Commented=on  w_specify=off  w_priority=None  w=0 rad/s  w_nominal_specify=off  w_nominal_unit=rad/s  w_nominal_value=1
      settings: SourceProductName=Foundation Library
  Solver Configuration : nesl_utility/Solver Configuration  PhysicalDomain=network_engine_domain  SubClassName=solver  LeftPortType=input  RightPortType=generic  Tones=[]  Harmonics=[]  SimulateNoise=off  Profile=off  UseLocalSampling=off  EnableSwitchedLinearOptims=on  FrequencyDomain=off  UseCCode=off  RelTol=0.001  AbsTol=1e-06  MinStep=1e-09  ToWorkspace=off  RevertToSquareIcSolve=off  NumHomotopyIterations=100  PhasorMode=off  PhasorModeNumHarmonics=1  DoDC=off  ResidualTolerance=1e-9  UseLocalSolver=off  LocalSolverChoice=NE_BACKWARD_EULER_ADVANCER  LocalSolverSampleTime=.001  DoFixedCost=off  MaxNonlinIter=3  MaxModeIter=2  ComputeImpulses=off  ImpulseIterations=2  LinearAlgebra=Sparse  EquationFormulation=NE_TIME_EF  DelaysMemoryBudget=1024  AutomaticFiltering=on  FilteringTimeConstant=0.001  PartitionStorageMethod=AS_NEEDED  PartitionMemoryBudget=1024  PartitionMethod=FAST
  Stepper Motor : ee_lib/Electromechanical/Reluctance & Stepper/Stepper Motor  step_or_av=1  w_vec=[ 0 1000 3e+3 ] rpm  T_vec=[ 2 2 1 ] N*m  assert_if_slipping=0  J_total=1e-4 kg*m^2  max_step_rate=10 Hz  Ra=0.55 Ohm  La=0.0015 H  Km=0.19 N*m/A  Td=0 N*m  Rm=Inf Ohm  Step=1.8 deg  J=4.5e-05 kg*m^2  B=1e-02 N*m/(rad/s)  speed0=0 rpm  theta0=0 deg  alpha=[.00393, .00393] 1/K  Tmeas=25 degC  T_vec2=[1.7, 1.7, .85] N*m  Tmeas2=125 degC  half_stepping=0  thermal_masses=[100, 100] J/K  initial_temperatures=[25, 25] degC  rotor_thermal_mass=50 J/K  rotor_initial_temperature=25 degC  Rm_percent_rotor=90
      settings: SourceProductBaseCode=PS  InternalSimscapePortConfiguration="{\"Bottom\":[],\"Left\":[{\"id\":\"pA\",\"label\":\"A+\",\"type\":\"foundation.electrical.electrical\"},{\"id\":\"nA\",\"label\":\"A-\",\"type\":\"foundation.electrical.electrical\"},{\"id\":\"pB\",\"label\":\"B+\",\"type\":\"foundation.electrical.electrical\"},{\"id\":\"nB\",\"label\":\"B-\",\"type\":\"foundation.electrical.electrical\"}],\"Right\":[{\"id\":\"R\",\"label\":\"R\",\"type\":\"foundation.mechanical.rotational.rotational\"},{\"id\":\"C\",\"label\":\"C\",\"type\":\"foundation.mechanical.rotational.rotational\"}],\"Top\":[]}"
  Stepper Motor Driver : ee_lib/Electromechanical/Reluctance & Stepper/Stepper Motor Driver  step_or_av=1  step_rate_per_volt=10 Hz/V  Venable=2.5 V  Vreverse=2.5 V  Vm=5 V  half_stepping=0
      settings: SourceProductBaseCode=PS  InternalSimscapePortConfiguration="{\"Bottom\":[],\"Left\":[{\"id\":\"ENA\",\"label\":\"ENA\",\"type\":\"foundation.electrical.electrical\"},{\"id\":\"REF\",\"label\":\"REF\",\"type\":\"foundation.electrical.electrical\"},{\"id\":\"REV\",\"label\":\"REV\",\"type\":\"foundation.electrical.electrical\"}],\"Right\":[{\"id\":\"pA\",\"label\":\"A+\",\"type\":\"foundation.electrical.electrical\"},{\"id\":\"nA\",\"label\":\"A-\",\"type\":\"foundation.electrical.electrical\"},{\"id\":\"pB\",\"label\":\"B+\",\"type\":\"foundation.electrical.electrical\"},{\"id\":\"nB\",\"label\":\"B-\",\"type\":\"foundation.electrical.electrical\"}],\"Top\":[]}"
  Motion : Outport
  wires:
  In1/1 -> Fcn/1
  Fcn/1 -> Controller/1(Ref)
  Constant1/1 -> Degrees to Radians/1
  PS-Simulink Converter/1 -> Add/1
  Degrees to Radians/1 -> Add/2
  Add/1 -> Motion/1
  connections:
  ERef B-/LConn1 -- Stepper Motor Driver/RConn4 -- Stepper Motor/LConn4
  Sensing Angle/LConn1 -- Shaft Free End/LConn1 -- Stepper Motor/RConn1
  MRRef Motor/LConn1 -- Stepper Motor/RConn2 -- Solver Configuration/RConn1
  ERef A+/LConn1 -- Stepper Motor Driver/RConn1 -- Stepper Motor/LConn1
  Stepper Motor Driver/RConn3 -- Stepper Motor/LConn3
  Stepper Motor/LConn2 -- Stepper Motor Driver/RConn2
  Controller/RConn1 -- Stepper Motor Driver/LConn1
  Controller/RConn2 -- Stepper Motor Driver/LConn2
  Controller/RConn3 -- Stepper Motor Driver/LConn3
  Sensing Angle/RConn1 -- PS-Simulink Converter/LConn1

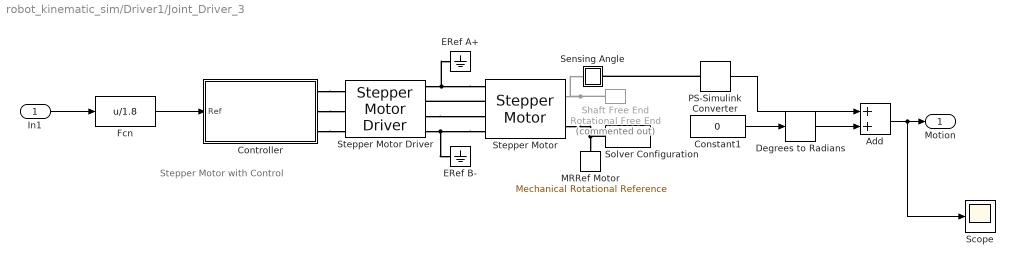
== system robot_kinematic_sim/Driver1/Joint_Driver_3 ==
  note: Stepper Motor with Control
  In1 : Inport
  Add : Sum
  Constant1 : Constant  Value=0
  Controller : Subsystem  Variant=on  VariantControlMode=label  LabelModeActiveChoice=Position
      settings: AttributesFormatString=%<BlockChoice>
  Degrees to Radians : simulink_extras/Transformations/Degrees to Radians
  ERef A+ : fl_lib/Electrical/Electrical Elements/Electrical Reference
      settings: SourceProductName=Foundation Library
  ERef B- : fl_lib/Electrical/Electrical Elements/Electrical Reference
      settings: SourceProductName=Foundation Library
  Fcn : Fcn  Expr=u/1.8
  MRRef Motor : fl_lib/Mechanical/Rotational Elements/Mechanical Rotational Reference
      settings: SourceProductName=Foundation Library
  PS-Simulink Converter : nesl_utility/PS-Simulink Converter  PhysicalDomain=network_engine_domain  SubClassName=ps_output  LeftPortType=input  RightPortType=output  PseudoPeriodic=off  Frequencies=[]  Unit=1  AffineConversion=off
  Scope : Scope
  Sensing Angle : Subsystem
      mask Display: disp('a')
  Shaft Free End : fl_lib/Mechanical/Rotational Elements/Rotational Free End  Commented=on  w_specify=off  w_priority=None  w=0 rad/s  w_nominal_specify=off  w_nominal_unit=rad/s  w_nominal_value=1
      settings: SourceProductName=Foundation Library
  Solver Configuration : nesl_utility/Solver Configuration  PhysicalDomain=network_engine_domain  SubClassName=solver  LeftPortType=input  RightPortType=generic  Tones=[]  Harmonics=[]  SimulateNoise=off  Profile=off  UseLocalSampling=off  EnableSwitchedLinearOptims=on  FrequencyDomain=off  UseCCode=off  RelTol=0.001  AbsTol=1e-06  MinStep=1e-09  ToWorkspace=off  RevertToSquareIcSolve=off  NumHomotopyIterations=100  PhasorMode=off  PhasorModeNumHarmonics=1  DoDC=off  ResidualTolerance=1e-9  UseLocalSolver=off  LocalSolverChoice=NE_BACKWARD_EULER_ADVANCER  LocalSolverSampleTime=.001  DoFixedCost=off  MaxNonlinIter=3  MaxModeIter=2  ComputeImpulses=off  ImpulseIterations=2  LinearAlgebra=Sparse  EquationFormulation=NE_TIME_EF  DelaysMemoryBudget=1024  AutomaticFiltering=on  FilteringTimeConstant=0.001  PartitionStorageMethod=AS_NEEDED  PartitionMemoryBudget=1024  PartitionMethod=FAST
  Stepper Motor : ee_lib/Electromechanical/Reluctance & Stepper/Stepper Motor  step_or_av=1  w_vec=[ 0 1000 3e+3 ] rpm  T_vec=[ 2 2 1 ] N*m  assert_if_slipping=0  J_total=1e-4 kg*m^2  max_step_rate=10 Hz  Ra=0.55 Ohm  La=0.0015 H  Km=0.19 N*m/A  Td=0 N*m  Rm=Inf Ohm  Step=1.8 deg  J=4.5e-05 kg*m^2  B=1e-02 N*m/(rad/s)  speed0=0 rpm  theta0=0 deg  alpha=[.00393, .00393] 1/K  Tmeas=25 degC  T_vec2=[1.7, 1.7, .85] N*m  Tmeas2=125 degC  half_stepping=0  thermal_masses=[100, 100] J/K  initial_temperatures=[25, 25] degC  rotor_thermal_mass=50 J/K  rotor_initial_temperature=25 degC  Rm_percent_rotor=90
      settings: SourceProductBaseCode=PS  InternalSimscapePortConfiguration="{\"Bottom\":[],\"Left\":[{\"id\":\"pA\",\"label\":\"A+\",\"type\":\"foundation.electrical.electrical\"},{\"id\":\"nA\",\"label\":\"A-\",\"type\":\"foundation.electrical.electrical\"},{\"id\":\"pB\",\"label\":\"B+\",\"type\":\"foundation.electrical.electrical\"},{\"id\":\"nB\",\"label\":\"B-\",\"type\":\"foundation.electrical.electrical\"}],\"Right\":[{\"id\":\"R\",\"label\":\"R\",\"type\":\"foundation.mechanical.rotational.rotational\"},{\"id\":\"C\",\"label\":\"C\",\"type\":\"foundation.mechanical.rotational.rotational\"}],\"Top\":[]}"
  Stepper Motor Driver : ee_lib/Electromechanical/Reluctance & Stepper/Stepper Motor Driver  step_or_av=1  step_rate_per_volt=10 Hz/V  Venable=2.5 V  Vreverse=2.5 V  Vm=5 V  half_stepping=0
      settings: SourceProductBaseCode=PS  InternalSimscapePortConfiguration="{\"Bottom\":[],\"Left\":[{\"id\":\"ENA\",\"label\":\"ENA\",\"type\":\"foundation.electrical.electrical\"},{\"id\":\"REF\",\"label\":\"REF\",\"type\":\"foundation.electrical.electrical\"},{\"id\":\"REV\",\"label\":\"REV\",\"type\":\"foundation.electrical.electrical\"}],\"Right\":[{\"id\":\"pA\",\"label\":\"A+\",\"type\":\"foundation.electrical.electrical\"},{\"id\":\"nA\",\"label\":\"A-\",\"type\":\"foundation.electrical.electrical\"},{\"id\":\"pB\",\"label\":\"B+\",\"type\":\"foundation.electrical.electrical\"},{\"id\":\"nB\",\"label\":\"B-\",\"type\":\"foundation.electrical.electrical\"}],\"Top\":[]}"
  Motion : Outport
  wires:
  Fcn/1 -> Controller/1(Ref)
  In1/1 -> Fcn/1
  Constant1/1 -> Degrees to Radians/1
  PS-Simulink Converter/1 -> Add/1
  Degrees to Radians/1 -> Add/2
  Add/1 -> Scope/1, Motion/1
  connections:
  PS-Simulink Converter/LConn1 -- Sensing Angle/RConn1
  Controller/RConn3 -- Stepper Motor Driver/LConn3
  Controller/RConn2 -- Stepper Motor Driver/LConn2
  Controller/RConn1 -- Stepper Motor Driver/LConn1
  Stepper Motor/LConn2 -- Stepper Motor Driver/RConn2
  Stepper Motor Driver/RConn3 -- Stepper Motor/LConn3
  Stepper Motor/LConn1 -- Stepper Motor Driver/RConn1 -- ERef A+/LConn1
  Solver Configuration/RConn1 -- Stepper Motor/RConn2 -- MRRef Motor/LConn1
  Shaft Free End/LConn1 -- Sensing Angle/LConn1 -- Stepper Motor/RConn1
  Stepper Motor/LConn4 -- Stepper Motor Driver/RConn4 -- ERef B-/LConn1

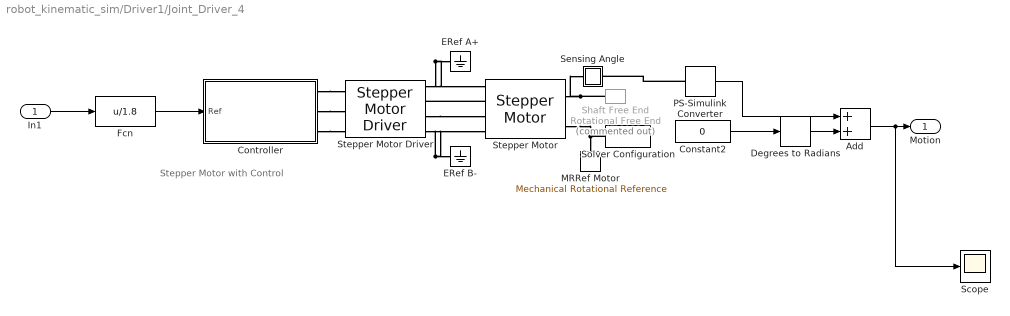
== system robot_kinematic_sim/Driver1/Joint_Driver_4 ==
  note: Stepper Motor with Control
  In1 : Inport
  Add : Sum
  Constant2 : Constant  Value=0
  Controller : Subsystem  Variant=on  VariantControlMode=label  LabelModeActiveChoice=Position
      settings: AttributesFormatString=%<BlockChoice>
  Degrees to Radians : simulink_extras/Transformations/Degrees to Radians
  ERef A+ : fl_lib/Electrical/Electrical Elements/Electrical Reference
      settings: SourceProductName=Foundation Library
  ERef B- : fl_lib/Electrical/Electrical Elements/Electrical Reference
      settings: SourceProductName=Foundation Library
  Fcn : Fcn  Expr=u/1.8
  MRRef Motor : fl_lib/Mechanical/Rotational Elements/Mechanical Rotational Reference
      settings: SourceProductName=Foundation Library
  PS-Simulink Converter : nesl_utility/PS-Simulink Converter  PhysicalDomain=network_engine_domain  SubClassName=ps_output  LeftPortType=input  RightPortType=output  PseudoPeriodic=off  Frequencies=[]  Unit=1  AffineConversion=off
  Scope : Scope
  Sensing Angle : Subsystem
      mask Display: disp('a')
  Shaft Free End : fl_lib/Mechanical/Rotational Elements/Rotational Free End  Commented=on  w_specify=off  w_priority=None  w=0 rad/s  w_nominal_specify=off  w_nominal_unit=rad/s  w_nominal_value=1
      settings: SourceProductName=Foundation Library
  Solver Configuration : nesl_utility/Solver Configuration  PhysicalDomain=network_engine_domain  SubClassName=solver  LeftPortType=input  RightPortType=generic  Tones=[]  Harmonics=[]  SimulateNoise=off  Profile=off  UseLocalSampling=off  EnableSwitchedLinearOptims=on  FrequencyDomain=off  UseCCode=off  RelTol=0.001  AbsTol=1e-06  MinStep=1e-09  ToWorkspace=off  RevertToSquareIcSolve=off  NumHomotopyIterations=100  PhasorMode=off  PhasorModeNumHarmonics=1  DoDC=off  ResidualTolerance=1e-9  UseLocalSolver=off  LocalSolverChoice=NE_BACKWARD_EULER_ADVANCER  LocalSolverSampleTime=.001  DoFixedCost=off  MaxNonlinIter=3  MaxModeIter=2  ComputeImpulses=off  ImpulseIterations=2  LinearAlgebra=Sparse  EquationFormulation=NE_TIME_EF  DelaysMemoryBudget=1024  AutomaticFiltering=on  FilteringTimeConstant=0.001  PartitionStorageMethod=AS_NEEDED  PartitionMemoryBudget=1024  PartitionMethod=FAST
  Stepper Motor : ee_lib/Electromechanical/Reluctance & Stepper/Stepper Motor  step_or_av=1  w_vec=[ 0 1000 3e+3 ] rpm  T_vec=[ 2 2 1 ] N*m  assert_if_slipping=0  J_total=1e-4 kg*m^2  max_step_rate=10 Hz  Ra=0.55 Ohm  La=0.0015 H  Km=0.19 N*m/A  Td=0 N*m  Rm=Inf Ohm  Step=1.8 deg  J=4.5e-05 kg*m^2  B=1e-02 N*m/(rad/s)  speed0=0 rpm  theta0=0 deg  alpha=[.00393, .00393] 1/K  Tmeas=25 degC  T_vec2=[1.7, 1.7, .85] N*m  Tmeas2=125 degC  half_stepping=0  thermal_masses=[100, 100] J/K  initial_temperatures=[25, 25] degC  rotor_thermal_mass=50 J/K  rotor_initial_temperature=25 degC  Rm_percent_rotor=90
      settings: SourceProductBaseCode=PS  InternalSimscapePortConfiguration="{\"Bottom\":[],\"Left\":[{\"id\":\"pA\",\"label\":\"A+\",\"type\":\"foundation.electrical.electrical\"},{\"id\":\"nA\",\"label\":\"A-\",\"type\":\"foundation.electrical.electrical\"},{\"id\":\"pB\",\"label\":\"B+\",\"type\":\"foundation.electrical.electrical\"},{\"id\":\"nB\",\"label\":\"B-\",\"type\":\"foundation.electrical.electrical\"}],\"Right\":[{\"id\":\"R\",\"label\":\"R\",\"type\":\"foundation.mechanical.rotational.rotational\"},{\"id\":\"C\",\"label\":\"C\",\"type\":\"foundation.mechanical.rotational.rotational\"}],\"Top\":[]}"
  Stepper Motor Driver : ee_lib/Electromechanical/Reluctance & Stepper/Stepper Motor Driver  step_or_av=1  step_rate_per_volt=10 Hz/V  Venable=2.5 V  Vreverse=2.5 V  Vm=5 V  half_stepping=0
      settings: SourceProductBaseCode=PS  InternalSimscapePortConfiguration="{\"Bottom\":[],\"Left\":[{\"id\":\"ENA\",\"label\":\"ENA\",\"type\":\"foundation.electrical.electrical\"},{\"id\":\"REF\",\"label\":\"REF\",\"type\":\"foundation.electrical.electrical\"},{\"id\":\"REV\",\"label\":\"REV\",\"type\":\"foundation.electrical.electrical\"}],\"Right\":[{\"id\":\"pA\",\"label\":\"A+\",\"type\":\"foundation.electrical.electrical\"},{\"id\":\"nA\",\"label\":\"A-\",\"type\":\"foundation.electrical.electrical\"},{\"id\":\"pB\",\"label\":\"B+\",\"type\":\"foundation.electrical.electrical\"},{\"id\":\"nB\",\"label\":\"B-\",\"type\":\"foundation.electrical.electrical\"}],\"Top\":[]}"
  Motion : Outport
  wires:
  In1/1 -> Fcn/1
  Fcn/1 -> Controller/1(Ref)
  Constant2/1 -> Degrees to Radians/1
  PS-Simulink Converter/1 -> Add/1
  Degrees to Radians/1 -> Add/2
  Add/1 -> Scope/1, Motion/1
  connections:
  ERef B-/LConn1 -- Stepper Motor Driver/RConn4 -- Stepper Motor/LConn4
  Stepper Motor/RConn1 -- Sensing Angle/LConn1 -- Shaft Free End/LConn1
  MRRef Motor/LConn1 -- Stepper Motor/RConn2 -- Solver Configuration/RConn1
  ERef A+/LConn1 -- Stepper Motor Driver/RConn1 -- Stepper Motor/LConn1
  Stepper Motor Driver/RConn3 -- Stepper Motor/LConn3
  Stepper Motor/LConn2 -- Stepper Motor Driver/RConn2
  Controller/RConn1 -- Stepper Motor Driver/LConn1
  Controller/RConn2 -- Stepper Motor Driver/LConn2
  Controller/RConn3 -- Stepper Motor Driver/LConn3
  PS-Simulink Converter/LConn1 -- Sensing Angle/RConn1
